simulink model "TosAeb"  (R2021a)
settings: Solver=FixedStepDiscrete  StartTime=0.0  StopTime=inf  FixedStep=0.01  RelTol=1e-3
undefined symbols (not defined in the file or in its folder): ACCModSts, AccSts1, AEB_ObjectMovingOrientation, AEB_ObjectMovingState, CN_BTCActive, CN_CurrentGearR, CN_CurrentGearR_DHT, CN_PTCActive, CN_VDCActive, CN_VSC_EspOff, CN_VSC_FCW_AEB_Error, CN_VSC_FCW_AEB_NoDispay, CN_VSC_FCW_AEB_PerfmDgdtn, CN_VSC_FCW_AEB_TempUnAvlbl, CN_VSC_VehStandstill, CNaccelAvoidMax, CNaccelAvoidMin, CNbrakePedalPress, CNcomfortStrLeftAccelMax, CNcomfortStrLeftAccelMin, CNcomfortStrRightAccelMax, CNcomfortStrRightAccelMin, CNDrvDoorOpen, CNDrvModeEco, CNDrvModeNorm, CNDrvModeOther, CNDrvModeSport, CNDrvSBRreminder, CNNull, CNobjInvalidID, CNPassDoorOpen, CNPassSBRreminder, CNPEBSAccelNegInf, CNPEBSAccelNull, CNPEBSTimeInf, CNPEBSTimeNull, CNSTOPMAX, CNSTOPNULL, CNsubjWidth, CNSwtOFF, CNSwtON, CNTTCMAX, CNTTCNULL, CNVehConfigAEBCycNone, CNVehConfigAEBNone, CNVehConfigAEBPedNone, CNVehConfigFCWNone, CNVehConfigJANone, CollisionSeverity, FALSE, FCWSnvty, JACycObjCalcInfoDefaultParams, JAPedObjCalcInfoDefaultParams, JAVehObjCalcInfoDefaultParams, latCycObjCalcInfoDefaultParams, latPedObjCalcInfoDefaultParams, longObjCalcInfoDefaultParams, ModSts, movingStatus, ObjectType ... (+1112)
libraries: simulink x3031, AEBSFunctionLib x50, Ta_Library x33, AEBSStateMachineLib x23, Mylibrary x12, simulink_extras x7, ObjSelect_lib x3, dspstat3 x2
(part 61 of 102)

== system TosAeb/FcwAebJa_Step_sys/ALG_AEBSystem/ALG_AEBController/AEB_FuncImpManagementApp/FuncManeuArbCalc/brkFuncManeuArbCalc/axTgtReqValueCalc1/axTgtReqValueCalc4AebControl/axTgtReqValueCalc/axTgtReqValueFinal1 ==
  axTgtReqValueK1 : Inport
  JerkFuncInc : Inport  Port=2
  JerkFuncDec : Inport  Port=3
  aDesired : Inport  Port=4
  accelMax : Inport  Port=5
  accelMin : Inport  Port=6
  Action Port : ActionPort  ActionPortLabel=if(u1)
  Constant : Constant  Value=Ts
  axTgtReqValueCalc : Subsystem  TreatAsAtomicUnit=on
  axTgtReqValue : Outport
  wires:
  JerkFuncInc/1 -> axTgtReqValueCalc/1(JerkFuncInc)
  axTgtReqValueK1/1 -> axTgtReqValueCalc/5(axTgtReqValueK1)
  JerkFuncDec/1 -> axTgtReqValueCalc/2(JerkFuncDec)
  axTgtReqValueCalc/1(axTgtReqValue) -> axTgtReqValue/1
  accelMin/1 -> axTgtReqValueCalc/7(accelMin)
  aDesired/1 -> axTgtReqValueCalc/4(aDesired)
  Constant/1 -> axTgtReqValueCalc/3(Ts)
  accelMax/1 -> axTgtReqValueCalc/6(accelMax)

== system TosAeb/FcwAebJa_Step_sys/ALG_AEBSystem/ALG_AEBController/AEB_FuncImpManagementApp/FuncManeuArbCalc/brkFuncManeuArbCalc/axTgtReqValueCalc1/axTgtReqValueCalc4AebControl/axTgtReqValueCalc/velReduceFlgCalc ==
  vx : Inport
  subjLongAccel : Inport  Port=2
  axTgtReqValueK1 : Inport  Port=3
  Compare To Constant : simulink/Logic and Bit Operations/Compare To Constant  relop=>  const=CNNull  OutDataTypeStr=boolean
  If : If  IfExpression=u1
  If Action Subsystem : Subsystem  TreatAsAtomicUnit=on
  If Action Subsystem1 : Subsystem  TreatAsAtomicUnit=on
  Merge : Merge
  Merge1 : Merge
  velReduceFlg : Outport
  axTgtReqValueK1Filt : Outport  Port=2
  wires:
  Merge1/1 -> axTgtReqValueK1Filt/1
  Merge/1 -> velReduceFlg/1
  If Action Subsystem1/2(axTgtReqValueK1Filt) -> Merge1/2
  If Action Subsystem/2(axTgtReqValueK1Filt) -> Merge1/1
  If Action Subsystem1/1(velReduceFlg) -> Merge/2
  If/2 -> If Action Subsystem1/Action
  axTgtReqValueK1/1 -> If Action Subsystem1/1(axTgtReqValueK1)
  subjLongAccel/1 -> If Action Subsystem/1(subjLongAccel)
  If/1 -> If Action Subsystem/Action
  Compare To Constant/1 -> If/1
  If Action Subsystem/1(velReduceFlg) -> Merge/1
  vx/1 -> Compare To Constant/1

== system TosAeb/FcwAebJa_Step_sys/ALG_AEBSystem/ALG_AEBController/AEB_JaObjCalcApp/JAPedTgtInfoCalc/JAPedCollisonAssessInfo/LatPedCollisonAssessInfoCalc/PedDistBrakeFullCalc/DistPhase2Calc/distPhase2Calc ==
  tPhase2 : Inport  OutDataTypeStr=single
  tPhase1 : Inport  Port=2  OutDataTypeStr=single
  subjLongAccelFilt : Inport  Port=3  OutDataTypeStr=single
  Kjerk : Inport  Port=4  OutDataTypeStr=single
  subjVel : Inport  Port=5  OutDataTypeStr=single
  Add : Sum  Inputs=4  OutDataTypeStr=single
  Constant : Constant  Value=1/6  OutDataTypeStr=single
  Constant1 : Constant  Value=0.5  OutDataTypeStr=single
  Product : Product  Inputs=5  OutDataTypeStr=single
  Product1 : Product  Inputs=4  OutDataTypeStr=single
  Product2 : Product  OutDataTypeStr=single
  Product3 : Product  Inputs=3  OutDataTypeStr=single
  distPhase2 : Outport
  wires:
  tPhase2/1 -> Product1/3, Product/1, Product/2, Product/3, Product2/2, Product3/3, Product1/2
  Kjerk/1 -> Product/4
  Constant/1 -> Product/5
  Constant1/1 -> Product1/1
  Product1/1 -> Add/1
  Add/1 -> distPhase2/1
  subjLongAccelFilt/1 -> Product3/1, Product1/4
  tPhase1/1 -> Product3/2
  subjVel/1 -> Product2/1
  Product3/1 -> Add/2
  Product2/1 -> Add/3
  Product/1 -> Add/4

== system TosAeb/FcwAebJa_Step_sys/ALG_AEBSystem/ALG_AEBController/AEB_JaObjCalcApp/JAPedTgtInfoCalc/JAPedCollisonAssessInfo/LatPedCollisonAssessInfoCalc/PedDistBrakeFullCalc/DistPhase2Calc/tPhase2Calc ==
  note: t jerk_amax = Max[0 ，（ a m ax - a ego ） /K jerk ] ；
  note: t jerk_0 = （ -a ego /K jer k ） + sqrt （（ a ego /K jer k ） ² - 2* （ v ego / K jer k ））
  note: t jerk_0 = （ -a ego /K jer k ） + sqrt （（ a ego /K jer k ） ² + 2* （ v ego / K jer k ））
  subjVel : Inport  OutDataTypeStr=single
  Kjerk : Inport  Port=2  OutDataTypeStr=single
  subjLongAccelFilt : Inport  Port=3  OutDataTypeStr=single
  accelMax : Inport  Port=4  OutDataTypeStr=single
  Add : Sum  Inputs=+-  OutDataTypeStr=single
  Add1 : Sum  Inputs=-+  OutDataTypeStr=single
  Add2 : Sum  Inputs=+-  OutDataTypeStr=single
  Constant : Constant  Value=0  OutDataTypeStr=single
  Divide : Product  Inputs=*/  OutDataTypeStr=single
  Divide1 : Product  Inputs=*/  OutDataTypeStr=single
  Divide2 : Product  Inputs=*/  OutDataTypeStr=single
  Gain : Gain  Gain=2  OutDataTypeStr=single
  Math Function : Math  Operator=square  SignedPower=on  OutDataTypeStr=single  SaturateOnIntegerOverflow=on
  MinMax : MinMax  Function=max  Inputs=2  OutDataTypeStr=single
  MinMax1 : MinMax  Inputs=2  OutDataTypeStr=single
  Relational Operator : RelationalOperator  Operator=<=  OutDataTypeStr=boolean  RndMeth=Simplest
  Sqrt : Sqrt  OutDataTypeStr=single  SaturateOnIntegerOverflow=on
  egoStopFlg : Outport
  tJerkWork : Outport  Port=2
  tPhase2 : Outport  Port=3
  wires:
  Add2/1 -> Divide2/1
  MinMax/1 -> tJerkWork/1, Relational Operator/2, MinMax1/1  "tJerkWork"
  subjLongAccelFilt/1 -> Add2/2, Divide/1
  Sqrt/1 -> Add1/2
  Kjerk/1 -> Divide2/2, Divide/2, Divide1/2
  accelMax/1 -> Add2/1
  Divide2/1 -> MinMax/1
  Constant/1 -> MinMax/2
  Divide/1 -> Add1/1, Math Function/1
  MinMax1/1 -> tPhase2/1
  Divide1/1 -> Gain/1
  Add/1 -> Sqrt/1
  Gain/1 -> Add/2
  Add1/1 -> Relational Operator/1, MinMax1/2  "tEgoStop"
  Math Function/1 -> Add/1
  subjVel/1 -> Divide1/1
  Relational Operator/1 -> egoStopFlg/1

== system TosAeb/FcwAebJa_Step_sys/ALG_AEBSystem/ALG_AEBController/AEB_JaObjCalcApp/JAPedTgtInfoCalc/JAPedCollisonAssessInfo/LatPedCollisonAssessInfoCalc/PedDistBrakeFullCalc/DistPhase3Calc/distPhase3Calc ==
  note: d3=（vego+ aego*（t1 + t2）+ 0.5* Kjerk* t2²）*t3 + 0.5* amax* t3²；
  tPhase3 : Inport  OutDataTypeStr=single
  subjVel : Inport  Port=2  OutDataTypeStr=single
  subjLongAccelFilt : Inport  Port=3  OutDataTypeStr=single
  Kjerk : Inport  Port=4  OutDataTypeStr=single
  tPhase1 : Inport  Port=5  OutDataTypeStr=single
  tPhase2 : Inport  Port=6  OutDataTypeStr=single
  accelMax : Inport  Port=7  OutDataTypeStr=single
  Add : Sum  OutDataTypeStr=single
  Add1 : Sum  Inputs=+++  OutDataTypeStr=single
  Add2 : Sum  OutDataTypeStr=single
  Constant1 : Constant  Value=0.5  OutDataTypeStr=single
  Constant2 : Constant  Value=0.5  OutDataTypeStr=single
  Product : Product  OutDataTypeStr=single
  Product1 : Product  Inputs=4  OutDataTypeStr=single
  Product2 : Product  OutDataTypeStr=single
  Product3 : Product  Inputs=4  OutDataTypeStr=single
  distPhase3 : Outport
  wires:
  tPhase1/1 -> Add/1
  tPhase2/1 -> Product1/2, Product1/1, Add/2
  Constant1/1 -> Product1/4
  Kjerk/1 -> Product1/3
  Product/1 -> Add1/2
  Product1/1 -> Add1/3
  subjVel/1 -> Add1/1
  Add1/1 -> Product2/2
  tPhase3/1 -> Product2/1, Product3/4, Product3/3
  Constant2/1 -> Product3/1
  accelMax/1 -> Product3/2
  Product3/1 -> Add2/1
  Product2/1 -> Add2/2
  Add2/1 -> distPhase3/1
  subjLongAccelFilt/1 -> Product/1
  Add/1 -> Product/2

== system TosAeb/FcwAebJa_Step_sys/ALG_AEBSystem/ALG_AEBController/AEB_JaObjCalcApp/JAPedTgtInfoCalc/JAPedCollisonAssessInfo/LatPedCollisonAssessInfoCalc/PedDistBrakeFullCalc/DistPhase3Calc/tPhase3Calc ==
  subjVel : Inport  OutDataTypeStr=single
  subjLongAccelFilt : Inport  Port=2  OutDataTypeStr=single
  tJerkWork : Inport  Port=3  OutDataTypeStr=single
  Kjerk : Inport  Port=4  OutDataTypeStr=single
  accelMax : Inport  Port=5  OutDataTypeStr=single
  velDelta : Inport  Port=6  OutDataTypeStr=single
  Action Port : ActionPort  ActionPortLabel=else
  Bus Creator : BusCreator  Inputs=6
  Bus Selector : BusSelector  OutputSignals=velDelta,subjLongAccelFilt,tJerkWork,Kjerk,accelMax
  Bus Selector1 : BusSelector  OutputSignals=subjVel,subjLongAccelFilt,tJerkWork,Kjerk,accelMax
  tEgoStopCalc : Subsystem
  tPhase3Calc : Subsystem
  tVelDeltaCalc : Subsystem
  tPhase3 : Outport
  wires:
  tVelDeltaCalc/1(tVelDelta) -> tPhase3Calc/1(tVelDelta)
  tEgoStopCalc/1(tEgoStop) -> tPhase3Calc/2(tEgoStop)
  tPhase3Calc/1(tPhase3) -> tPhase3/1
  subjVel/1 -> Bus Creator/1  "subjVel"
  subjLongAccelFilt/1 -> Bus Creator/2  "subjLongAccelFilt"
  tJerkWork/1 -> Bus Creator/3  "tJerkWork"
  Kjerk/1 -> Bus Creator/4  "Kjerk"
  accelMax/1 -> Bus Creator/5  "accelMax"
  velDelta/1 -> Bus Creator/6  "velDelta"
  Bus Creator/1 -> Bus Selector1/1, Bus Selector/1
  Bus Selector/3 -> tVelDeltaCalc/3(tJerkWork)  "<tJerkWork>"
  Bus Selector/2 -> tVelDeltaCalc/2(subjLongAccelFilt)  "<subjLongAccelFilt>"
  Bus Selector/4 -> tVelDeltaCalc/4(Kjerk)  "<Kjerk>"
  Bus Selector/1 -> tVelDeltaCalc/1(velDelta)  "<velDelta>"
  Bus Selector/5 -> tVelDeltaCalc/5(accelMax)  "<accelMax>"
  Bus Selector1/1 -> tEgoStopCalc/1(subjVel)  "<subjVel>"
  Bus Selector1/2 -> tEgoStopCalc/2(subjLongAccelFilt)  "<subjLongAccelFilt>"
  Bus Selector1/3 -> tEgoStopCalc/3(tJerkWork)  "<tJerkWork>"
  Bus Selector1/4 -> tEgoStopCalc/4(Kjerk)  "<Kjerk>"
  Bus Selector1/5 -> tEgoStopCalc/5(accelMax)  "<accelMax>"

== system TosAeb/FcwAebJa_Step_sys/ALG_AEBSystem/ALG_AEBController/AEB_JaObjCalcApp/JAPedTgtInfoCalc/JAPedCollisonAssessInfo/LatPedCollisonAssessInfoCalc/PedDistBrakeFullCalc/DistPhase3Calc/tPhase3Calc1 ==
  Action Port : ActionPort  ActionPortLabel=if(u1)
  Constant : Constant  Value=0  OutDataTypeStr=single
  tPhase3 : Outport
  wires:
  Constant/1 -> tPhase3/1

== system TosAeb/FcwAebJa_Step_sys/ALG_AEBSystem/ALG_AEBController/AEB_JaObjCalcApp/JAPedTgtInfoCalc/JAPedObjCalcInfoCalc/JAPedObjInfoCalc/ObjCrossFlgCal/CollisionProbCalc/If Action Subsystem ==
  Action Port : ActionPort  ActionPortLabel=if(u1)
  Constant : Constant  Value=0  OutDataTypeStr=boolean
  ObjColliFlg : Outport
  wires:
  Constant/1 -> ObjColliFlg/1

== system TosAeb/FcwAebJa_Step_sys/ALG_AEBSystem/ALG_AEBController/AEB_JaObjCalcApp/JAPedTgtInfoCalc/JAPedObjCalcInfoCalc/JAPedObjInfoCalc/ObjCrossFlgCal/CollisionProbCalc/ObjIsInvalidCalc ==
  ObjectID : Inport
  Compare To Constant : simulink/Logic and Bit Operations/Compare To Constant  relop===  const=CNobjInvalidID  OutDataTypeStr=boolean
  ObjIsInvalid : Outport
  wires:
  Compare To Constant/1 -> ObjIsInvalid/1
  ObjectID/1 -> Compare To Constant/1

== system TosAeb/FcwAebJa_Step_sys/ALG_AEBSystem/ALG_AEBController/AEB_JaObjCalcApp/JAPedTgtInfoCalc/JAPedObjCalcInfoCalc/JAPedObjInfoCalc/ObjCrossFlgCal/CollisionProbCalc/Subsystem ==
  tPredTime : Inport  OutDataTypeStr=single
  subjVel : Inport  Port=2  OutDataTypeStr=single
  subjLongAccel : Inport  Port=3  OutDataTypeStr=single
  subjLatAccel : Inport  Port=4  OutDataTypeStr=single
  subjYawRate : Inport  Port=5  OutDataTypeStr=single
  ObjectYawAngleCorr : Inport  Port=6  OutDataTypeStr=single
  ObjectMovingOrientation : Inport  Port=7
  ObjectLength : Inport  Port=8  OutDataTypeStr=single
  ObjectTypeCameraConfirmed : Inport  Port=9
  ObjectWidth : Inport  Port=10  OutDataTypeStr=single
  dx : Inport  Port=11  OutDataTypeStr=single
  dy : Inport  Port=12  OutDataTypeStr=single
  vyRelCorr : Inport  Port=13  OutDataTypeStr=single
  vxAbsObj : Inport  Port=14  OutDataTypeStr=single
  Action Port : ActionPort  ActionPortLabel=else
  Gain : Gain
  ObjBoundingBox : Subsystem
  ObjLenthWidthCalc : Subsystem
  ObjPreReferPointCalc : Subsystem
  PreCrossFlgCalcFlow : Stateflow chart  TreatAsAtomicUnit=on
  PreRefPointEgoCalc : Subsystem
  SubjBoundingBox : Subsystem
  Terminator : Terminator
  sincosCoefCalc : Subsystem
  sincosCoefCalc1 : Subsystem
  ObjColliFlg : Outport
  wires:
  subjYawRate/1 -> PreRefPointEgoCalc/5(subjYawRate)
  subjLatAccel/1 -> PreRefPointEgoCalc/4(subjLatAccel), [subjLatAccel]
  subjVel/1 -> PreRefPointEgoCalc/1(subjVel)
  subjLongAccel/1 -> PreRefPointEgoCalc/3(subjLongAccel)
  vxAbsObj/1 -> ObjPreReferPointCalc/2(vxAbsObj)
  dx/1 -> ObjPreReferPointCalc/1(dx)
  ObjectWidth/1 -> ObjLenthWidthCalc/5(ObjectWidth)
  ObjectLength/1 -> ObjLenthWidthCalc/3(ObjectLength)
  ObjectTypeCameraConfirmed/1 -> ObjLenthWidthCalc/4(ObjectTypeCameraConfirmed)
  PreCrossFlgCalcFlow/1(preCrossFlg) -> ObjColliFlg/1
  ObjBoundingBox/2(objDyPre) -> PreCrossFlgCalcFlow/4(objDyPre)
  ObjBoundingBox/1(objDxPre) -> PreCrossFlgCalcFlow/3(objDxPre)
  SubjBoundingBox/2(subjDyPre) -> PreCrossFlgCalcFlow/2(subjDyPre)
  SubjBoundingBox/1(subjDxPre) -> PreCrossFlgCalcFlow/1(subjDxPre)
  sincosCoefCalc1/1(sincosCoef) -> ObjBoundingBox/5(sincosCoef)
  ObjPreReferPointCalc/2(dyPreRefPointObj) -> ObjBoundingBox/4(dyPreRefPointObj)
  ObjPreReferPointCalc/1(dxPreRefPointObj) -> ObjBoundingBox/3(dxPreRefPointObj)
  ObjLenthWidthCalc/1(ObjLengthCorr) -> ObjBoundingBox/1(ObjLengthCorr)
  ObjLenthWidthCalc/2(ObjWidthCorr) -> ObjBoundingBox/2(ObjWidthCorr)
  ObjPreReferPointCalc/3(YawAnglePreObj) -> sincosCoefCalc1/1(angle)
  PreRefPointEgoCalc/1(" dxPreRefPoint") -> SubjBoundingBox/1(dxPreRefPoint)
  sincosCoefCalc/1(sincosCoef) -> SubjBoundingBox/3(sincosCoef)
  PreRefPointEgoCalc/2(" dyPreRefPoint") -> SubjBoundingBox/2(dyPreRefPoint)
  PreRefPointEgoCalc/3(YawAnglePre) -> sincosCoefCalc/1(angle)
  dy/1 -> ObjPreReferPointCalc/5(dy)
  vyRelCorr/1 -> ObjPreReferPointCalc/4(vyRelCorr)
  ObjectMovingOrientation/1 -> ObjLenthWidthCalc/2(ObjectMovingOrientation)
  tPredTime/1 -> ObjPreReferPointCalc/3(tPredTime), PreRefPointEgoCalc/2(tPredTime)
  ObjectYawAngleCorr/1 -> ObjLenthWidthCalc/1(ObjectYawAngle), ObjPreReferPointCalc/6(ObjectYawAngleCorr)
  Gain/1 -> Terminator/1
  [subjLatAccel] -> Gain/1

== system TosAeb/FcwAebJa_Step_sys/ALG_AEBSystem/ALG_AEBController/AEB_JaObjCalcApp/JAVehTgtInfoCalc/AEBJAVehObjCalc/JAVehObjInfoCalc/ObjCrossFlgCal/CollisionProbCalc/If Action Subsystem ==
  Action Port : ActionPort  ActionPortLabel=if(u1 == 0)
  Constant : Constant  Value=0  OutDataTypeStr=boolean
  ObjColliFlg : Outport
  wires:
  Constant/1 -> ObjColliFlg/1

== system TosAeb/FcwAebJa_Step_sys/ALG_AEBSystem/ALG_AEBController/AEB_JaObjCalcApp/JAVehTgtInfoCalc/AEBJAVehObjCalc/JAVehObjInfoCalc/ObjCrossFlgCal/CollisionProbCalc/Subsystem ==
  tPredTime : Inport  OutDataTypeStr=single
  subjVel : Inport  Port=2
  subjLongAccel : Inport  Port=3
  subjLatAccel : Inport  Port=4
  subjYawRate : Inport  Port=5
  ObjectYawAngle : Inport  Port=6  OutDataTypeStr=single
  ObjectMovingOrientation : Inport  Port=7
  ObjectLength : Inport  Port=8  OutDataTypeStr=single
  FusionType : Inport  Port=9
  ObjectWidth : Inport  Port=10  OutDataTypeStr=single
  dx : Inport  Port=11  OutDataTypeStr=single
  vy : Inport  Port=12  OutDataTypeStr=single
  ay : Inport  Port=13  OutDataTypeStr=single
  dy : Inport  Port=14  OutDataTypeStr=single
  vxAbsObj : Inport  Port=15  OutDataTypeStr=single
  axAbsObj : Inport  Port=16  OutDataTypeStr=single
  Action Port : ActionPort  ActionPortLabel=else
  ObjBoundingBoxRear : Subsystem
  ObjBoundingBoxfront : Subsystem  Commented=on
  ObjLenthWidthCalc : Subsystem
  ObjPreReferPointCalc : Subsystem
  PreCrossFlgCalcFlow : Stateflow chart  TreatAsAtomicUnit=on
  PreRefPointEgoCalc : Subsystem
  SubjBoundingBox : Subsystem
  sincosCoefCalc : Subsystem
  sincosCoefCalc1 : Subsystem
  ObjColliFlg : Outport
  wires:
  [subjLatAccel] -> ObjPreReferPointCalc/6(subjLatAccel)
  ay/1 -> ObjPreReferPointCalc/7(ay)
  dy/1 -> ObjPreReferPointCalc/8(dy)
  [tPredTime] -> ObjPreReferPointCalc/3(tPredTime)
  subjYawRate/1 -> PreRefPointEgoCalc/5(subjYawRate)
  subjLatAccel/1 -> [subjLatAccel], PreRefPointEgoCalc/4(subjLatAccel)
  subjVel/1 -> PreRefPointEgoCalc/1(subjVel)
  subjLongAccel/1 -> PreRefPointEgoCalc/3(subjLongAccel)
  tPredTime/1 -> [tPredTime], PreRefPointEgoCalc/2(tPredTime)
  vy/1 -> ObjPreReferPointCalc/5(vy)
  axAbsObj/1 -> ObjPreReferPointCalc/4(axAbsObj)
  vxAbsObj/1 -> ObjPreReferPointCalc/2(vxAbsObj)
  dx/1 -> ObjPreReferPointCalc/1(dx)
  ObjectYawAngle/1 -> ObjPreReferPointCalc/9(ObjectYawAngle), ObjLenthWidthCalc/1(ObjectYawAngle)
  ObjectWidth/1 -> ObjLenthWidthCalc/5(ObjectWidth)
  ObjectLength/1 -> ObjLenthWidthCalc/3(ObjectLength)
  FusionType/1 -> ObjLenthWidthCalc/4(FusionType)
  PreCrossFlgCalcFlow/1(preCrossFlg) -> ObjColliFlg/1
  ObjBoundingBoxRear/2(objDyPre) -> PreCrossFlgCalcFlow/4(objDyPre)
  ObjBoundingBoxRear/1(objDxPre) -> PreCrossFlgCalcFlow/3(objDxPre)
  SubjBoundingBox/2(subjDyPre) -> PreCrossFlgCalcFlow/2(subjDyPre)
  SubjBoundingBox/1(subjDxPre) -> PreCrossFlgCalcFlow/1(subjDxPre)
  sincosCoefCalc1/1(sincosCoef) -> ObjBoundingBoxRear/5(sincosCoef)
  ObjPreReferPointCalc/2(dyPreRefPointObj) -> ObjBoundingBoxRear/4(dyPreRefPointObj)
  ObjPreReferPointCalc/1(dxPreRefPointObj) -> ObjBoundingBoxRear/3(dxPreRefPointObj)
  ObjLenthWidthCalc/1(ObjLengthCorr) -> ObjBoundingBoxRear/1(ObjLengthCorr)
  ObjLenthWidthCalc/2(ObjWidthCorr) -> ObjBoundingBoxRear/2(ObjWidthCorr)
  ObjPreReferPointCalc/3(YawAnglePreObj) -> sincosCoefCalc1/1(angle)
  PreRefPointEgoCalc/1(" dxPreRefPoint") -> SubjBoundingBox/1(dxPreRefPoint)
  sincosCoefCalc/1(sincosCoef) -> SubjBoundingBox/3(sincosCoef)
  PreRefPointEgoCalc/2(" dyPreRefPoint") -> SubjBoundingBox/2(dyPreRefPoint)
  PreRefPointEgoCalc/3(YawAnglePre) -> sincosCoefCalc/1(angle)
  ObjectMovingOrientation/1 -> ObjLenthWidthCalc/2(ObjectMovingOrientation)

== system TosAeb/FcwAebJa_Step_sys/ALG_AEBSystem/ALG_AEBController/AEB_JaObjCalcApp/JAVehTgtInfoCalc/JAVehCollisonAssessInfoCalc/JaVehCollisonAssessInfoCalc/VehDistBrakeFullCalc/DistPhase2Calc/distPhase2Calc ==
  note: d2= （ vego+ aego*t1 ） *t2 + 0.5* aego* t2² + 1/6* Kjerk * t2 ³
  tPhase2 : Inport  OutDataTypeStr=single
  subjAccelFilt : Inport  Port=2  OutDataTypeStr=single
  Kjerk : Inport  Port=3  OutDataTypeStr=single
  subjVel : Inport  Port=4  OutDataTypeStr=single
  Add : Sum  Inputs=3  OutDataTypeStr=single
  Constant : Constant  Value=1/6  OutDataTypeStr=single
  Constant1 : Constant  Value=0.5  OutDataTypeStr=single
  Product : Product  Inputs=5  OutDataTypeStr=single
  Product1 : Product  Inputs=4  OutDataTypeStr=single
  Product2 : Product  OutDataTypeStr=single
  distPhase2 : Outport
  wires:
  tPhase2/1 -> Product1/3, Product1/4, Product2/2, Product/1, Product/2, Product/3
  Kjerk/1 -> Product/4
  Constant/1 -> Product/5
  subjAccelFilt/1 -> Product1/2
  Constant1/1 -> Product1/1
  subjVel/1 -> Product2/1
  Product2/1 -> Add/2
  Product/1 -> Add/3
  Product1/1 -> Add/1
  Add/1 -> distPhase2/1

== system TosAeb/FcwAebJa_Step_sys/ALG_AEBSystem/ALG_AEBController/AEB_JaObjCalcApp/JAVehTgtInfoCalc/JAVehCollisonAssessInfoCalc/JaVehCollisonAssessInfoCalc/VehDistBrakeFullCalc/DistPhase2Calc/tPhase2Calc ==
  note: change '-' to '+'
  note: t jerk_0 = （ -a ego /K jer k ） + sqrt （（ a ego /K jer k ） ²+ 2* （ v ego / K jer k ））
  note: t jerk_amax = Max[0 ，（ a m ax - a ego ） /K jerk ] ；
  note: t jerk_0 = （ -a ego /K jer k ） + sqrt （（ a ego /K jer k ） ²- 2* （ v ego / K jer k ））
  subjVel : Inport  OutDataTypeStr=single
  Kjerk : Inport  Port=2  OutDataTypeStr=single
  subjLongAccelFilt : Inport  Port=3  OutDataTypeStr=single
  accelMax : Inport  Port=4  OutDataTypeStr=single
  Add : Sum  Inputs=+-  OutDataTypeStr=single
  Add1 : Sum  Inputs=-+  OutDataTypeStr=single
  Add2 : Sum  Inputs=+-  OutDataTypeStr=single
  Constant : Constant  Value=0  OutDataTypeStr=single
  Divide : Product  Inputs=*/  OutDataTypeStr=single
  Divide1 : Product  Inputs=*/  OutDataTypeStr=single
  Divide2 : Product  Inputs=*/  OutDataTypeStr=single
  Gain : Gain  Gain=2  OutDataTypeStr=single
  Math Function : Math  Operator=square  SignedPower=on  OutDataTypeStr=single  SaturateOnIntegerOverflow=on
  MinMax : MinMax  Function=max  Inputs=2  OutDataTypeStr=single
  MinMax1 : MinMax  Inputs=2  OutDataTypeStr=single
  Relational Operator : RelationalOperator  Operator=<=  OutDataTypeStr=boolean  RndMeth=Simplest
  Sqrt : Sqrt  OutDataTypeStr=single  SaturateOnIntegerOverflow=on
  egoStopFlg : Outport
  tJerkWork : Outport  Port=2
  tPhase2 : Outport  Port=3
  wires:
  Add2/1 -> Divide2/1
  MinMax/1 -> tJerkWork/1, Relational Operator/2, MinMax1/1  "tJerkWork"
  subjLongAccelFilt/1 -> Add2/2, Divide/1
  Sqrt/1 -> Add1/2
  Kjerk/1 -> Divide2/2, Divide/2, Divide1/2
  accelMax/1 -> Add2/1
  Divide2/1 -> MinMax/1
  Constant/1 -> MinMax/2
  Divide/1 -> Add1/1, Math Function/1
  MinMax1/1 -> tPhase2/1
  Divide1/1 -> Gain/1
  Add/1 -> Sqrt/1
  Gain/1 -> Add/2
  Add1/1 -> Relational Operator/1, MinMax1/2  "tEgoStop"
  Math Function/1 -> Add/1
  subjVel/1 -> Divide1/1
  Relational Operator/1 -> egoStopFlg/1

== system TosAeb/FcwAebJa_Step_sys/ALG_AEBSystem/ALG_AEBController/AEB_JaObjCalcApp/JAVehTgtInfoCalc/JAVehCollisonAssessInfoCalc/JaVehCollisonAssessInfoCalc/VehDistBrakeFullCalc/DistPhase3Calc/distPhase3Calc ==
  note: d3=（vego+aego*（t1 + t2）+ 0.5* Kjerk* t2²）*t3 + 0.5* amax* t3²；
  tPhase3 : Inport  OutDataTypeStr=single
  subjVel : Inport  Port=2  OutDataTypeStr=single
  subjLongAccelFilt : Inport  Port=3  OutDataTypeStr=single
  Kjerk : Inport  Port=4  OutDataTypeStr=single
  tPhase1 : Inport  Port=5  OutDataTypeStr=single
  tPhase2 : Inport  Port=6  OutDataTypeStr=single
  accelMax : Inport  Port=7  OutDataTypeStr=single
  Add : Sum  OutDataTypeStr=single
  Add1 : Sum  Inputs=+++  OutDataTypeStr=single
  Add2 : Sum  OutDataTypeStr=single
  Constant1 : Constant  Value=0.5  OutDataTypeStr=single
  Constant2 : Constant  Value=0.5  OutDataTypeStr=single
  Product : Product  OutDataTypeStr=single
  Product1 : Product  Inputs=4  OutDataTypeStr=single
  Product2 : Product  OutDataTypeStr=single
  Product3 : Product  Inputs=4  OutDataTypeStr=single
  distPhase3 : Outport
  wires:
  tPhase1/1 -> Add/1
  tPhase2/1 -> Product1/2, Product1/1, Add/2
  Add/1 -> Product/2
  Constant1/1 -> Product1/4
  Kjerk/1 -> Product1/3
  Product/1 -> Add1/2
  Product1/1 -> Add1/3
  subjVel/1 -> Add1/1
  Add1/1 -> Product2/2
  tPhase3/1 -> Product2/1, Product3/4, Product3/3
  Constant2/1 -> Product3/1
  accelMax/1 -> Product3/2
  Product3/1 -> Add2/1
  Product2/1 -> Add2/2
  Add2/1 -> distPhase3/1
  subjLongAccelFilt/1 -> Product/1

== system TosAeb/FcwAebJa_Step_sys/ALG_AEBSystem/ALG_AEBController/AEB_JaObjCalcApp/JAVehTgtInfoCalc/JAVehCollisonAssessInfoCalc/JaVehCollisonAssessInfoCalc/VehDistBrakeFullCalc/DistPhase3Calc/tPhase3Calc ==
  subjVel : Inport  OutDataTypeStr=single
  subjLongAccelFilt : Inport  Port=2  OutDataTypeStr=single
  tJerkWork : Inport  Port=3  OutDataTypeStr=single
  Kjerk : Inport  Port=4  OutDataTypeStr=single
  accelMax : Inport  Port=5  OutDataTypeStr=single
  velDelta : Inport  Port=6  OutDataTypeStr=single
  Action Port : ActionPort  ActionPortLabel=else
  Bus Creator : BusCreator  Inputs=6
  Bus Selector : BusSelector  OutputSignals=velDelta,subjLongAccelFilt,tJerkWork,Kjerk,accelMax
  Bus Selector1 : BusSelector  OutputSignals=subjVel,subjLongAccelFilt,tJerkWork,Kjerk,accelMax
  tEgoStopCalc : Subsystem
  tPhase3Calc : Subsystem
  tVelDeltaCalc : Subsystem
  tPhase3 : Outport
  wires:
  tEgoStopCalc/1(tEgoStop) -> tPhase3Calc/2(tEgoStop)
  tPhase3Calc/1(tPhase3) -> tPhase3/1
  subjVel/1 -> Bus Creator/1  "subjVel"
  subjLongAccelFilt/1 -> Bus Creator/2  "subjLongAccelFilt"
  tJerkWork/1 -> Bus Creator/3  "tJerkWork"
  Kjerk/1 -> Bus Creator/4  "Kjerk"
  accelMax/1 -> Bus Creator/5  "accelMax"
  velDelta/1 -> Bus Creator/6  "velDelta"
  Bus Creator/1 -> Bus Selector1/1, Bus Selector/1
  Bus Selector/3 -> tVelDeltaCalc/3(tJerkWork)  "<tJerkWork>"
  Bus Selector/2 -> tVelDeltaCalc/2(subjLongAccelFilt)  "<subjLongAccelFilt>"
  Bus Selector/4 -> tVelDeltaCalc/4(Kjerk)  "<Kjerk>"
  Bus Selector/1 -> tVelDeltaCalc/1(velDelta)  "<velDelta>"
  Bus Selector/5 -> tVelDeltaCalc/5(accelMax)  "<accelMax>"
  Bus Selector1/1 -> tEgoStopCalc/1(subjVel)  "<subjVel>"
  Bus Selector1/2 -> tEgoStopCalc/2(subjLongAccelFilt)  "<subjLongAccelFilt>"
  Bus Selector1/3 -> tEgoStopCalc/3(tJerkWork)  "<tJerkWork>"
  Bus Selector1/4 -> tEgoStopCalc/4(Kjerk)  "<Kjerk>"
  Bus Selector1/5 -> tEgoStopCalc/5(accelMax)  "<accelMax>"
  tVelDeltaCalc/1(tVelDelta) -> tPhase3Calc/1(tVelDelta)

== system TosAeb/FcwAebJa_Step_sys/ALG_AEBSystem/ALG_AEBController/AEB_JaObjCalcApp/JAVehTgtInfoCalc/JAVehCollisonAssessInfoCalc/JaVehCollisonAssessInfoCalc/VehDistBrakeFullCalc/DistPhase3Calc/tPhase3Calc1 ==
  Action Port : ActionPort  ActionPortLabel=if(u1)
  Constant : Constant  Value=0  OutDataTypeStr=single
  tPhase3 : Outport
  wires:
  Constant/1 -> tPhase3/1

== system TosAeb/FcwAebJa_Step_sys/ALG_AEBSystem/ALG_AEBController/AEB_JaObjCalcApp/JaCycTgtInfoCalc/JaCycCollisonAssessInfo/JaCycCollisonAssessInfoCalc/PedDistBrakeFullCalc/DistPhase2Calc/distPhase2Calc ==
  tPhase2 : Inport  OutDataTypeStr=single
  tPhase1 : Inport  Port=2  OutDataTypeStr=single
  subjLongAccelFilt : Inport  Port=3  OutDataTypeStr=single
  Kjerk : Inport  Port=4  OutDataTypeStr=single
  subjVel : Inport  Port=5  OutDataTypeStr=single
  Add : Sum  Inputs=4  OutDataTypeStr=single
  Constant : Constant  Value=1/6  OutDataTypeStr=single
  Constant1 : Constant  Value=0.5  OutDataTypeStr=single
  Product : Product  Inputs=5  OutDataTypeStr=single
  Product1 : Product  Inputs=4  OutDataTypeStr=single
  Product2 : Product  OutDataTypeStr=single
  Product3 : Product  Inputs=3  OutDataTypeStr=single
  distPhase2 : Outport
  wires:
  tPhase2/1 -> Product1/3, Product/1, Product/2, Product/3, Product2/2, Product3/3, Product1/2
  Kjerk/1 -> Product/4
  Constant/1 -> Product/5
  Constant1/1 -> Product1/1
  Product1/1 -> Add/1
  Add/1 -> distPhase2/1
  subjLongAccelFilt/1 -> Product3/1, Product1/4
  tPhase1/1 -> Product3/2
  subjVel/1 -> Product2/1
  Product3/1 -> Add/2
  Product2/1 -> Add/3
  Product/1 -> Add/4

== system TosAeb/FcwAebJa_Step_sys/ALG_AEBSystem/ALG_AEBController/AEB_JaObjCalcApp/JaCycTgtInfoCalc/JaCycCollisonAssessInfo/JaCycCollisonAssessInfoCalc/PedDistBrakeFullCalc/DistPhase2Calc/tPhase2Calc ==
  note: t jerk_amax = Max[0 ，（ a m ax - a ego ） /K jerk ] ；
  note: t jerk_0 = （ -a ego /K jer k ） + sqrt （（ a ego /K jer k ） ² - 2* （ v ego / K jer k ））
  note: t jerk_0 = （ -a ego /K jer k ） + sqrt （（ a ego /K jer k ） ² + 2* （ v ego / K jer k ））
  subjVel : Inport  OutDataTypeStr=single
  Kjerk : Inport  Port=2  OutDataTypeStr=single
  subjLongAccelFilt : Inport  Port=3  OutDataTypeStr=single
  accelMax : Inport  Port=4  OutDataTypeStr=single
  Add : Sum  Inputs=+-  OutDataTypeStr=single
  Add1 : Sum  Inputs=-+  OutDataTypeStr=single
  Add2 : Sum  Inputs=+-  OutDataTypeStr=single
  Constant : Constant  Value=0  OutDataTypeStr=single
  Divide : Product  Inputs=*/  OutDataTypeStr=single
  Divide1 : Product  Inputs=*/  OutDataTypeStr=single
  Divide2 : Product  Inputs=*/  OutDataTypeStr=single
  Gain : Gain  Gain=2  OutDataTypeStr=single
  Math Function : Math  Operator=square  SignedPower=on  OutDataTypeStr=single  SaturateOnIntegerOverflow=on
  MinMax : MinMax  Function=max  Inputs=2  OutDataTypeStr=single
  MinMax1 : MinMax  Inputs=2  OutDataTypeStr=single
  Relational Operator : RelationalOperator  Operator=<=  OutDataTypeStr=boolean  RndMeth=Simplest
  Sqrt : Sqrt  OutDataTypeStr=single  SaturateOnIntegerOverflow=on
  egoStopFlg : Outport
  tJerkWork : Outport  Port=2
  tPhase2 : Outport  Port=3
  wires:
  Add2/1 -> Divide2/1
  MinMax/1 -> tJerkWork/1, Relational Operator/2, MinMax1/1  "tJerkWork"
  subjLongAccelFilt/1 -> Add2/2, Divide/1
  Sqrt/1 -> Add1/2
  Kjerk/1 -> Divide2/2, Divide/2, Divide1/2
  accelMax/1 -> Add2/1
  Divide2/1 -> MinMax/1
  Constant/1 -> MinMax/2
  Divide/1 -> Add1/1, Math Function/1
  MinMax1/1 -> tPhase2/1
  Divide1/1 -> Gain/1
  Add/1 -> Sqrt/1
  Gain/1 -> Add/2
  Add1/1 -> Relational Operator/1, MinMax1/2  "tEgoStop"
  Math Function/1 -> Add/1
  subjVel/1 -> Divide1/1
  Relational Operator/1 -> egoStopFlg/1

== system TosAeb/FcwAebJa_Step_sys/ALG_AEBSystem/ALG_AEBController/AEB_JaObjCalcApp/JaCycTgtInfoCalc/JaCycCollisonAssessInfo/JaCycCollisonAssessInfoCalc/PedDistBrakeFullCalc/DistPhase3Calc/distPhase3Calc ==
  note: d3=（vego+ aego*（t1 + t2）+ 0.5* Kjerk* t2²）*t3 + 0.5* amax* t3²；
  tPhase3 : Inport  OutDataTypeStr=single
  subjVel : Inport  Port=2  OutDataTypeStr=single
  subjLongAccelFilt : Inport  Port=3  OutDataTypeStr=single
  Kjerk : Inport  Port=4  OutDataTypeStr=single
  tPhase1 : Inport  Port=5  OutDataTypeStr=single
  tPhase2 : Inport  Port=6  OutDataTypeStr=single
  accelMax : Inport  Port=7  OutDataTypeStr=single
  Add : Sum  OutDataTypeStr=single
  Add1 : Sum  Inputs=+++  OutDataTypeStr=single
  Add2 : Sum  OutDataTypeStr=single
  Constant1 : Constant  Value=0.5  OutDataTypeStr=single
  Constant2 : Constant  Value=0.5  OutDataTypeStr=single
  Product : Product  OutDataTypeStr=single
  Product1 : Product  Inputs=4  OutDataTypeStr=single
  Product2 : Product  OutDataTypeStr=single
  Product3 : Product  Inputs=4  OutDataTypeStr=single
  distPhase3 : Outport
  wires:
  tPhase1/1 -> Add/1
  tPhase2/1 -> Product1/2, Product1/1, Add/2
  Constant1/1 -> Product1/4
  Kjerk/1 -> Product1/3
  Product/1 -> Add1/2
  Product1/1 -> Add1/3
  subjVel/1 -> Add1/1
  Add1/1 -> Product2/2
  tPhase3/1 -> Product2/1, Product3/4, Product3/3
  Constant2/1 -> Product3/1
  accelMax/1 -> Product3/2
  Product3/1 -> Add2/1
  Product2/1 -> Add2/2
  Add2/1 -> distPhase3/1
  subjLongAccelFilt/1 -> Product/1
  Add/1 -> Product/2

== system TosAeb/FcwAebJa_Step_sys/ALG_AEBSystem/ALG_AEBController/AEB_JaObjCalcApp/JaCycTgtInfoCalc/JaCycCollisonAssessInfo/JaCycCollisonAssessInfoCalc/PedDistBrakeFullCalc/DistPhase3Calc/tPhase3Calc ==
  subjVel : Inport  OutDataTypeStr=single
  subjLongAccelFilt : Inport  Port=2  OutDataTypeStr=single
  tJerkWork : Inport  Port=3  OutDataTypeStr=single
  Kjerk : Inport  Port=4  OutDataTypeStr=single
  accelMax : Inport  Port=5  OutDataTypeStr=single
  velDelta : Inport  Port=6  OutDataTypeStr=single
  Action Port : ActionPort  ActionPortLabel=else
  Bus Creator : BusCreator  Inputs=6
  Bus Selector : BusSelector  OutputSignals=velDelta,subjLongAccelFilt,tJerkWork,Kjerk,accelMax
  Bus Selector1 : BusSelector  OutputSignals=subjVel,subjLongAccelFilt,tJerkWork,Kjerk,accelMax
  tEgoStopCalc : Subsystem
  tPhase3Calc : Subsystem
  tVelDeltaCalc : Subsystem
  tPhase3 : Outport
  wires:
  tVelDeltaCalc/1(tVelDelta) -> tPhase3Calc/1(tVelDelta)
  tEgoStopCalc/1(tEgoStop) -> tPhase3Calc/2(tEgoStop)
  tPhase3Calc/1(tPhase3) -> tPhase3/1
  subjVel/1 -> Bus Creator/1  "subjVel"
  subjLongAccelFilt/1 -> Bus Creator/2  "subjLongAccelFilt"
  tJerkWork/1 -> Bus Creator/3  "tJerkWork"
  Kjerk/1 -> Bus Creator/4  "Kjerk"
  accelMax/1 -> Bus Creator/5  "accelMax"
  velDelta/1 -> Bus Creator/6  "velDelta"
  Bus Creator/1 -> Bus Selector1/1, Bus Selector/1
  Bus Selector/3 -> tVelDeltaCalc/3(tJerkWork)  "<tJerkWork>"
  Bus Selector/2 -> tVelDeltaCalc/2(subjLongAccelFilt)  "<subjLongAccelFilt>"
  Bus Selector/4 -> tVelDeltaCalc/4(Kjerk)  "<Kjerk>"
  Bus Selector/1 -> tVelDeltaCalc/1(velDelta)  "<velDelta>"
  Bus Selector/5 -> tVelDeltaCalc/5(accelMax)  "<accelMax>"
  Bus Selector1/1 -> tEgoStopCalc/1(subjVel)  "<subjVel>"
  Bus Selector1/2 -> tEgoStopCalc/2(subjLongAccelFilt)  "<subjLongAccelFilt>"
  Bus Selector1/3 -> tEgoStopCalc/3(tJerkWork)  "<tJerkWork>"
  Bus Selector1/4 -> tEgoStopCalc/4(Kjerk)  "<Kjerk>"
  Bus Selector1/5 -> tEgoStopCalc/5(accelMax)  "<accelMax>"

== system TosAeb/FcwAebJa_Step_sys/ALG_AEBSystem/ALG_AEBController/AEB_JaObjCalcApp/JaCycTgtInfoCalc/JaCycCollisonAssessInfo/JaCycCollisonAssessInfoCalc/PedDistBrakeFullCalc/DistPhase3Calc/tPhase3Calc1 ==
  Action Port : ActionPort  ActionPortLabel=if(u1)
  Constant : Constant  Value=0  OutDataTypeStr=single
  tPhase3 : Outport
  wires:
  Constant/1 -> tPhase3/1

== system TosAeb/FcwAebJa_Step_sys/ALG_AEBSystem/ALG_AEBController/AEB_JaObjCalcApp/JaCycTgtInfoCalc/JaCycObjCalcInfoCalc/JaCycObjInfoCalc/ObjCrossFlgCal/CollisionProbCalc/If Action Subsystem ==
  Action Port : ActionPort  ActionPortLabel=if(u1)
  Constant : Constant  Value=FALSE  OutDataTypeStr=boolean
  ObjColliFlg : Outport
  wires:
  Constant/1 -> ObjColliFlg/1

== system TosAeb/FcwAebJa_Step_sys/ALG_AEBSystem/ALG_AEBController/AEB_JaObjCalcApp/JaCycTgtInfoCalc/JaCycObjCalcInfoCalc/JaCycObjInfoCalc/ObjCrossFlgCal/CollisionProbCalc/ObjIsInvalidCalc ==
  ObjectID : Inport
  Compare To Constant : simulink/Logic and Bit Operations/Compare To Constant  relop===  const=CNobjInvalidID  OutDataTypeStr=boolean
  ObjIsInvalid : Outport
  wires:
  Compare To Constant/1 -> ObjIsInvalid/1
  ObjectID/1 -> Compare To Constant/1

== system TosAeb/FcwAebJa_Step_sys/ALG_AEBSystem/ALG_AEBController/AEB_JaObjCalcApp/JaCycTgtInfoCalc/JaCycObjCalcInfoCalc/JaCycObjInfoCalc/ObjCrossFlgCal/CollisionProbCalc/Subsystem ==
  tPredTime : Inport  OutDataTypeStr=single
  subjVel : Inport  Port=2  OutDataTypeStr=single
  subjLongAccel : Inport  Port=3  OutDataTypeStr=single
  subjLatAccel : Inport  Port=4  OutDataTypeStr=single
  subjYawRate : Inport  Port=5  OutDataTypeStr=single
  ObjectYawAngleCorr : Inport  Port=6  OutDataTypeStr=single
  ObjectTypeCameraConfirmed : Inport  Port=7
  ObjectLength : Inport  Port=8  OutDataTypeStr=single
  ObjectWidth : Inport  Port=9  OutDataTypeStr=single
  ObjectMovingOrientation : Inport  Port=10
  dx : Inport  Port=11  OutDataTypeStr=single
  dy : Inport  Port=12  OutDataTypeStr=single
  vyRelCorr : Inport  Port=13  OutDataTypeStr=single
  vxAbsObj : Inport  Port=14  OutDataTypeStr=single
  Action Port : ActionPort  ActionPortLabel=else
  ObjBoundingBox : Subsystem
  ObjLenthWidthCalc : Subsystem
  ObjPreReferPointCalc : Subsystem
  PreCrossFlgCalcFlow : Stateflow chart  TreatAsAtomicUnit=on
  PreRefPointEgoCalc : Subsystem
  SubjBoundingBox : Subsystem
  sincosCoefCalc : Subsystem
  sincosCoefCalc1 : Subsystem
  ObjColliFlg : Outport
  wires:
  subjYawRate/1 -> PreRefPointEgoCalc/5(subjYawRate)
  subjLatAccel/1 -> PreRefPointEgoCalc/4(subjLatAccel)
  subjVel/1 -> PreRefPointEgoCalc/1(subjVel)
  subjLongAccel/1 -> PreRefPointEgoCalc/3(subjLongAccel)
  vxAbsObj/1 -> ObjPreReferPointCalc/2(vxAbsObj)
  dx/1 -> ObjPreReferPointCalc/1(dx)
  ObjectYawAngleCorr/1 -> ObjLenthWidthCalc/1(ObjectYawAngleCorr), ObjPreReferPointCalc/6(ObjectYawObjectYawAngleCorrAngle)
  PreCrossFlgCalcFlow/1(preCrossFlg) -> ObjColliFlg/1
  ObjBoundingBox/2(objDyPre) -> PreCrossFlgCalcFlow/4(objDyPre)
  ObjBoundingBox/1(objDxPre) -> PreCrossFlgCalcFlow/3(objDxPre)
  SubjBoundingBox/2(subjDyPre) -> PreCrossFlgCalcFlow/2(subjDyPre)
  SubjBoundingBox/1(subjDxPre) -> PreCrossFlgCalcFlow/1(subjDxPre)
  sincosCoefCalc1/1(sincosCoef) -> ObjBoundingBox/5(sincosCoef)
  ObjPreReferPointCalc/2(dyPreRefPointObj) -> ObjBoundingBox/4(dyPreRefPointObj)
  ObjPreReferPointCalc/1(dxPreRefPointObj) -> ObjBoundingBox/3(dxPreRefPointObj)
  ObjLenthWidthCalc/1(ObjLengthCorr) -> ObjBoundingBox/1(ObjLengthCorr)
  ObjLenthWidthCalc/2(ObjWidthCorr) -> ObjBoundingBox/2(ObjWidthCorr)
  ObjPreReferPointCalc/3(YawAnglePreObj) -> sincosCoefCalc1/1(angle)
  PreRefPointEgoCalc/1(" dxPreRefPoint") -> SubjBoundingBox/1(dxPreRefPoint)
  sincosCoefCalc/1(sincosCoef) -> SubjBoundingBox/3(sincosCoef)
  PreRefPointEgoCalc/2(" dyPreRefPoint") -> SubjBoundingBox/2(dyPreRefPoint)
  PreRefPointEgoCalc/3(YawAnglePre) -> sincosCoefCalc/1(angle)
  dy/1 -> ObjPreReferPointCalc/5(dy)
  vyRelCorr/1 -> ObjPreReferPointCalc/4(vyRelCorr)
  ObjectMovingOrientation/1 -> ObjLenthWidthCalc/5(ObjectMovingOrientation)
  tPredTime/1 -> ObjPreReferPointCalc/3(tPredTime), PreRefPointEgoCalc/2(tPredTime)
  ObjectLength/1 -> ObjLenthWidthCalc/3(ObjectLength)
  ObjectWidth/1 -> ObjLenthWidthCalc/4(ObjectWidth)
  ObjectTypeCameraConfirmed/1 -> ObjLenthWidthCalc/2(ObjectTypeCameraConfirmed)

== system TosAeb/FcwAebJa_Step_sys/ALG_AEBSystem/ALG_AEBController/AEB_LatObjInfoCalcApp/LatCycObjCalc/LatCycCollisonAssessInfoCalc/LatCycCollisonAssessInfoCalc/DistBrkCondCalc/CycDistBrakeFullCalc/BrkSys ==
  subjVel : Inport
  subjLongAccel : Inport  Port=2  OutDataTypeStr=single
  BrkJerkMaxFullBrakeLookupTable : Lookup_n-D  NumberOfTableDimensions=1  BreakpointsForDimension1=P_AEB_LatCycCA_BrkJerkMaxFullBrakeX  Table=P_AEB_LatCycCA_BrkJerkMaxFullBrakeY  ExtrapMethod=Clip  InternalRulePriority=Speed  RndMeth=Simplest  InputSameDT=on
  Compare To Constant : simulink/Logic and Bit Operations/Compare To Constant  relop=>=  const=P_AEB_LatCycCA_VelReduceMaxVelThresFullBrake  OutDataTypeStr=boolean
  DecelMaxFullBrakeLookup : Lookup_n-D  NumberOfTableDimensions=1  BreakpointsForDimension1=P_AEB_LatCycCA_DecelMaxFullBrakeX  Table=P_AEB_LatCycCA_DecelMaxFullBrakeY  ExtrapMethod=Clip  InternalRulePriority=Speed  RndMeth=Simplest  InputSameDT=on
  MinMax : MinMax  Function=max  Inputs=2  OutDataTypeStr=single
  P_LatCycCA_BrkJerkMaxFullBrake : Constant  Commented=on  Value=P_AEB_LatCycCA_BrkJerkMaxFullBrake  OutDataTypeStr=single
  P_LatCycCA_BrkSysDelayTimeFullBrake : Constant  Value=P_AEB_LatCycCA_BrkSysDelayTimeFullBrake  OutDataTypeStr=single
  P_LatCycCA_DecelMaxFullBrake : Constant  Commented=on  Value=P_AEB_LatCycCA_DecelMaxFullBrake  OutDataTypeStr=single
  P_LatCycCA_VelReduceMaxFullBrake : Constant  Value=P_AEB_LatCycCA_VelReduceMaxFullBrake  OutDataTypeStr=single
  P_LatCycCA_VelReduceMaxOver40KphFullBrake : Constant  Value=P_AEB_LatCycCA_VelReduceMaxOver40KphFullBrake  OutDataTypeStr=single
  Switch : Switch  Criteria=u2 ~= 0  OutDataTypeStr=single
  tDelay : Outport
  Kjerk : Outport  Port=2
  velDelta : Outport  Port=3
  accelMax : Outport  Port=4
  subjLongAccelFilt : Outport  Port=5
  wires:
  P_LatCycCA_BrkSysDelayTimeFullBrake/1 -> tDelay/1
  Compare To Constant/1 -> Switch/2
  P_LatCycCA_VelReduceMaxOver40KphFullBrake/1 -> Switch/1
  P_LatCycCA_VelReduceMaxFullBrake/1 -> Switch/3
  BrkJerkMaxFullBrakeLookupTable/1 -> Kjerk/1
  Switch/1 -> velDelta/1
  subjVel/1 -> Compare To Constant/1, BrkJerkMaxFullBrakeLookupTable/1, DecelMaxFullBrakeLookup/1
  subjLongAccel/1 -> MinMax/2
  MinMax/1 -> subjLongAccelFilt/1
  DecelMaxFullBrakeLookup/1 -> MinMax/1, accelMax/1

== system TosAeb/FcwAebJa_Step_sys/ALG_AEBSystem/ALG_AEBController/AEB_LatObjInfoCalcApp/LatCycObjCalc/LatCycCollisonAssessInfoCalc/LatCycCollisonAssessInfoCalc/DistBrkCondCalc/CycDistBrakeFullCalc/DistPhase1Calc ==
  subjVel : Inport
  tPhase1 : Inport  Port=2  OutDataTypeStr=single
  subjLongAccelFilt : Inport  Port=3  OutDataTypeStr=single
  Add : Sum  OutDataTypeStr=single
  Constant : Constant  Value=0.5  OutDataTypeStr=single
  Product : Product  Inputs=4  OutDataTypeStr=single
  Product1 : Product  OutDataTypeStr=single
  distPhase1 : Outport
  wires:
  tPhase1/1 -> Product1/2, Product/2, Product/1
  subjLongAccelFilt/1 -> Product/3
  Constant/1 -> Product/4
  subjVel/1 -> Product1/1
  Product1/1 -> Add/1
  Product/1 -> Add/2
  Add/1 -> distPhase1/1

== system TosAeb/FcwAebJa_Step_sys/ALG_AEBSystem/ALG_AEBController/AEB_LatObjInfoCalcApp/LatCycObjCalc/LatCycCollisonAssessInfoCalc/LatCycCollisonAssessInfoCalc/DistBrkCondCalc/CycDistBrakeFullCalc/DistPhase2Calc ==
  subjVel : Inport
  Kjerk : Inport  Port=2  OutDataTypeStr=single
  subjLongAccelFilt : Inport  Port=3  OutDataTypeStr=single
  accelMax : Inport  Port=4  OutDataTypeStr=single
  distPhase2Calc : Subsystem
  tPhase2Calc : Subsystem
  distPhase2 : Outport
  egoStopFlg : Outport  Port=2
  tJerkWork : Outport  Port=3
  tPhase2 : Outport  Port=4
  wires:
  subjLongAccelFilt/1 -> tPhase2Calc/3(subjLongAccelFilt), distPhase2Calc/2(subjAccelFilt)
  Kjerk/1 -> tPhase2Calc/2(Kjerk), distPhase2Calc/3(Kjerk)
  subjVel/1 -> distPhase2Calc/4(subjVel), tPhase2Calc/1(subjVel)
  accelMax/1 -> tPhase2Calc/4(accelMax)
  tPhase2Calc/3(tPhase2) -> tPhase2/1, distPhase2Calc/1(tPhase2)
  distPhase2Calc/1(distPhase2) -> distPhase2/1
  tPhase2Calc/2(tJerkWork) -> tJerkWork/1
  tPhase2Calc/1(egoStopFlg) -> egoStopFlg/1

== system TosAeb/FcwAebJa_Step_sys/ALG_AEBSystem/ALG_AEBController/AEB_LatObjInfoCalcApp/LatCycObjCalc/LatCycCollisonAssessInfoCalc/LatCycCollisonAssessInfoCalc/DistBrkCondCalc/CycDistBrakeFullCalc/DistPhase3Calc ==
  egoStopFlg : Inport
  tJerkWork : Inport  Port=2  OutDataTypeStr=single
  tPhase2 : Inport  Port=3  OutDataTypeStr=single
  tPhase1 : Inport  Port=4  OutDataTypeStr=single
  velDelta : Inport  Port=5  OutDataTypeStr=single
  subjVel : Inport  Port=6
  subjLongAccelFilt : Inport  Port=7  OutDataTypeStr=single
  Kjerk : Inport  Port=8  OutDataTypeStr=single
  accelMax : Inport  Port=9  OutDataTypeStr=single
  Bus Creator : BusCreator  Inputs=8
  Bus Selector : BusSelector  OutputSignals=subjVel,subjLongAccelFilt,tJerkWork,Kjerk,accelMax,velDelta
  Bus Selector1 : BusSelector  OutputSignals=subjVel,subjLongAccelFilt,Kjerk,tPhase1,tPhase2,accelMax
  If : If  IfExpression=u1
  Merge : Merge
  distPhase3Calc : Subsystem
  tPhase3Calc : Subsystem  TreatAsAtomicUnit=on
  tPhase3Calc1 : Subsystem  TreatAsAtomicUnit=on
  distPhase3 : Outport
  wires:
  If/1 -> tPhase3Calc1/Action
  egoStopFlg/1 -> If/1
  If/2 -> tPhase3Calc/Action
  tPhase3Calc1/1(tPhase3) -> Merge/1
  tPhase3Calc/1(tPhase3) -> Merge/2
  Merge/1 -> distPhase3Calc/1(tPhase3)
  distPhase3Calc/1(distPhase3) -> distPhase3/1
  accelMax/1 -> Bus Creator/8  "accelMax"
  Kjerk/1 -> Bus Creator/7  "Kjerk"
  subjLongAccelFilt/1 -> Bus Creator/6  "subjLongAccelFilt"
  subjVel/1 -> Bus Creator/5  "subjVel"
  tJerkWork/1 -> Bus Creator/1  "tJerkWork"
  tPhase1/1 -> Bus Creator/2  "tPhase1"
  tPhase2/1 -> Bus Creator/3  "tPhase2"
  velDelta/1 -> Bus Creator/4  "velDelta"
  Bus Creator/1 -> Bus Selector1/1, Bus Selector/1
  Bus Selector/3 -> tPhase3Calc/3(tJerkWork)  "<tJerkWork>"
  Bus Selector/4 -> tPhase3Calc/4(Kjerk)  "<Kjerk>"
  Bus Selector/2 -> tPhase3Calc/2(subjLongAccelFilt)  "<subjLongAccelFilt>"
  Bus Selector/5 -> tPhase3Calc/5(accelMax)  "<accelMax>"
  Bus Selector/1 -> tPhase3Calc/1(subjVel)  "<subjVel>"
  Bus Selector/6 -> tPhase3Calc/6(velDelta)  "<velDelta>"
  Bus Selector1/6 -> distPhase3Calc/7(accelMax)  "<accelMax>"
  Bus Selector1/5 -> distPhase3Calc/6(tPhase2)  "<tPhase2>"
  Bus Selector1/4 -> distPhase3Calc/5(tPhase1)  "<tPhase1>"
  Bus Selector1/3 -> distPhase3Calc/4(Kjerk)  "<Kjerk>"
  Bus Selector1/2 -> distPhase3Calc/3(subjLongAccelFilt)  "<subjLongAccelFilt>"
  Bus Selector1/1 -> distPhase3Calc/2(subjVel)  "<subjVel>"

== system TosAeb/FcwAebJa_Step_sys/ALG_AEBSystem/ALG_AEBController/AEB_LatObjInfoCalcApp/LatCycObjCalc/LatCycCollisonAssessInfoCalc/LatCycCollisonAssessInfoCalc/DistBrkCondCalc/CycDistBrakeFullCalc/distBrakeCalc ==
  distPhase1 : Inport  OutDataTypeStr=single
  distPhase2 : Inport  Port=2  OutDataTypeStr=single
  distPhase3 : Inport  Port=3  OutDataTypeStr=single
  Add : Sum  Inputs=3  OutDataTypeStr=single
  distBrake : Outport
  wires:
  distPhase2/1 -> Add/2
  distPhase1/1 -> Add/1
  distPhase3/1 -> Add/3
  Add/1 -> distBrake/1

== system TosAeb/FcwAebJa_Step_sys/ALG_AEBSystem/ALG_AEBController/AEB_LatObjInfoCalcApp/LatCycObjCalc/LatCycCollisonAssessInfoCalc/LatCycCollisonAssessInfoCalc/DistBrkCondCalc/CycDistBrakePartCalc/BrkSys ==
  subjVel : Inport
  subjLongAccel : Inport  Port=2  OutDataTypeStr=single
  BrkJerkMaxPartBrake : Lookup_n-D  NumberOfTableDimensions=1  BreakpointsForDimension1=P_AEB_LatCycCA_BrkJerkMaxPartBrakeX  Table=P_AEB_LatCycCA_BrkJerkMaxPartBrakeY  ExtrapMethod=Clip  InternalRulePriority=Speed  RndMeth=Simplest  InputSameDT=on
  Compare To Constant : simulink/Logic and Bit Operations/Compare To Constant  relop=>=  const=P_AEB_LatCycCA_VelReduceMaxVelThresPartBrake  OutDataTypeStr=boolean
  DecelMaxPartBrakeLookup Table : Lookup_n-D  NumberOfTableDimensions=1  BreakpointsForDimension1=P_AEB_LatCycCA_DecelMaxPartBrakeX  Table=P_AEB_LatCycCA_DecelMaxPartBrakeY  ExtrapMethod=Clip  InternalRulePriority=Speed  RndMeth=Simplest  InputSameDT=on
  MinMax : MinMax  Function=max  Inputs=2  OutDataTypeStr=single
  P_LatCycCA_BrkJerkMaxPartBrake : Constant  Commented=on  Value=P_AEB_LatCycCA_BrkJerkMaxPartBrake  OutDataTypeStr=single
  P_LatCycCA_BrkSysDelayTimePartBrake : Constant  Value=P_AEB_LatCycCA_BrkSysDelayTimePartBrake  OutDataTypeStr=single
  P_LatCycCA_DecelMaxPartBrake : Constant  Commented=on  Value=P_AEB_LatCycCA_DecelMaxPartBrake  OutDataTypeStr=single
  P_LatCycCA_VelReduceMaxOver40KphPartBrake : Constant  Value=P_AEB_LatCycCA_VelReduceMaxOver40KphPartBrake  OutDataTypeStr=single
  P_LatCycCA_VelReduceMaxPartBrake : Constant  Value=P_AEB_LatCycCA_VelReduceMaxPartBrake  OutDataTypeStr=single
  Switch : Switch  Criteria=u2 ~= 0  OutDataTypeStr=single
  tDelay : Outport
  Kjerk : Outport  Port=2
  velDelta : Outport  Port=3
  accelMax : Outport  Port=4
  subjLongAccelFilt : Outport  Port=5
  wires:
  MinMax/1 -> subjLongAccelFilt/1
  subjLongAccel/1 -> MinMax/2
  subjVel/1 -> DecelMaxPartBrakeLookup Table/1, BrkJerkMaxPartBrake/1, Compare To Constant/1
  DecelMaxPartBrakeLookup Table/1 -> accelMax/1, MinMax/1
  Switch/1 -> velDelta/1
  BrkJerkMaxPartBrake/1 -> Kjerk/1
  P_LatCycCA_VelReduceMaxPartBrake/1 -> Switch/3
  P_LatCycCA_VelReduceMaxOver40KphPartBrake/1 -> Switch/1
  Compare To Constant/1 -> Switch/2
  P_LatCycCA_BrkSysDelayTimePartBrake/1 -> tDelay/1

== system TosAeb/FcwAebJa_Step_sys/ALG_AEBSystem/ALG_AEBController/AEB_LatObjInfoCalcApp/LatCycObjCalc/LatCycCollisonAssessInfoCalc/LatCycCollisonAssessInfoCalc/DistBrkCondCalc/CycDistBrakePartCalc/DistPhase1Calc ==
  subjVel : Inport
  tPhase1 : Inport  Port=2  OutDataTypeStr=single
  subjLongAccelFilt : Inport  Port=3  OutDataTypeStr=single
  Add : Sum  OutDataTypeStr=single
  Constant : Constant  Value=0.5  OutDataTypeStr=single
  Product : Product  Inputs=4  OutDataTypeStr=single
  Product1 : Product  OutDataTypeStr=single
  distPhase1 : Outport
  wires:
  Add/1 -> distPhase1/1
  Product/1 -> Add/2
  Product1/1 -> Add/1
  subjVel/1 -> Product1/1
  Constant/1 -> Product/4
  subjLongAccelFilt/1 -> Product/3
  tPhase1/1 -> Product/1, Product/2, Product1/2

== system TosAeb/FcwAebJa_Step_sys/ALG_AEBSystem/ALG_AEBController/AEB_LatObjInfoCalcApp/LatCycObjCalc/LatCycCollisonAssessInfoCalc/LatCycCollisonAssessInfoCalc/DistBrkCondCalc/CycDistBrakePartCalc/DistPhase2Calc ==
  subjVel : Inport
  Kjerk : Inport  Port=2  OutDataTypeStr=single
  subjLongAccelFilt : Inport  Port=3  OutDataTypeStr=single
  accelMax : Inport  Port=4  OutDataTypeStr=single
  distPhase2Calc : Subsystem
  tPhase2Calc : Subsystem
  distPhase2 : Outport
  egoStopFlg : Outport  Port=2
  tJerkWork : Outport  Port=3
  tPhase2 : Outport  Port=4
  wires:
  tPhase2Calc/1(egoStopFlg) -> egoStopFlg/1
  tPhase2Calc/2(tJerkWork) -> tJerkWork/1
  distPhase2Calc/1(distPhase2) -> distPhase2/1
  tPhase2Calc/3(tPhase2) -> distPhase2Calc/1(tPhase2), tPhase2/1
  accelMax/1 -> tPhase2Calc/4(accelMax)
  subjVel/1 -> tPhase2Calc/1(subjVel), distPhase2Calc/4(subjVel)
  Kjerk/1 -> distPhase2Calc/3(Kjerk), tPhase2Calc/2(Kjerk)
  subjLongAccelFilt/1 -> distPhase2Calc/2(subjAccelFilt), tPhase2Calc/3(subjLongAccelFilt)
